simulink library "LibrarySimulinkSASv2014b"  (R2014b)
settings: Solver=ode45  SolverMode=SingleTasking  StartTime=0.0  StopTime=10.0  RelTol=1e-3
undefined symbols (not defined in the file or in its folder): shmControl, ts
undefined symbols (not defined in the file): IdB, IdL, IdM
libraries: LibrarySimulinkSAS x15, $bdroot x1
(part 1 of 4)
settings Model: CovSaveCumulativeToWorkspaceVar=on  Creator=dsantiago  LastModifiedBy=dds

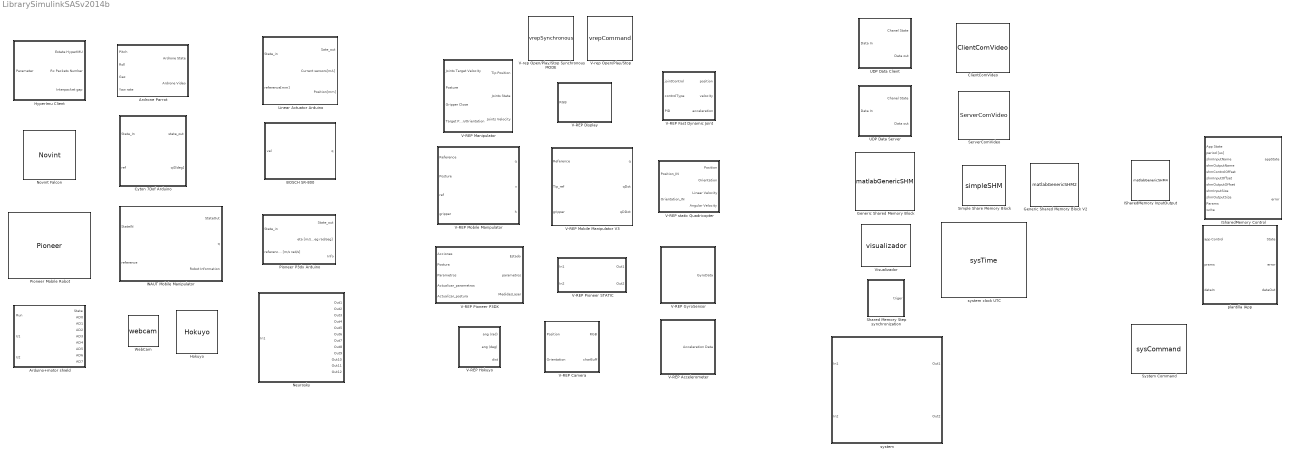
== system LibrarySimulinkSASv2014b ==
  Ardrone Parrot : Subsystem  mask{Dev='ARDRONE', ts=-1}
      mask prompts: Dev "Ardrone Parrot version"; ts "Sample Time"
      mask Help:
      | HELP DEVICE
      | 
      | - UDP Client for the server "ArdroneParrot.exe" App
      | 
      | HELP DRIVERS
      | 
      | -------------------------------------------------------------------------------------------------------------------------------------------------------
      | HELP APP
      | 
      | Before running the Simulink model initiate communication with the device through the application 'ardroneParrot.exe' available from folder '\SAS TOOLS\Software Ardrone Parrot V1.1.0'. After executing the program the console-type app is available.
      | 
      | 
      | -------------------------------------------------------------------------------------------------------------------------------------------------------
      | 
      | HELP BLOCK
      | 
      | Input Ports
      | 
      | Port 'Pitch reference': desde Matlab, entre -1 y 1, +-28.65 grados
      | Port 'Roll reference': desde Matlab, entre -1 y 1, +-28.65 grados
      | Port 'Gaz reference': desde Matlab, entre -1 y 1, +- 1 m/s
      | Port 'Yaw rate reference': desde Matlab, entre -1 y 1, +- 172 grados/segundo
      | 
      | Output Ports
      | 
      | Port 'State ArdroneParrot': sensors information from the Parrot quadcopter including a vector 'dispositivo' of 20 signals type doubles
      | 
      | dispositivo[0] -> Battery level (1 -> 100%)
      | dispositivo[1] -> Phi en rad (roll)
      | dispositivo[2] -> Theta en rad (pitch)
      | dispositivo[3] -> Psi en rad (yaw)
      | dispositivo[4] -> Altitud (en metros)
      | dispositivo[5] -> Velocidad eje X (forward) (en m/s)
      | dispositivo[6] -> Velocidad eje Y (sideward) (en m/s)
      | dispositivo[7] -> Velocidad eje Z (en m/s)
      | dispositivo[8] -> Giróscopo[0] (en deg/s)
      | dispositivo[9] -> Giróscopo[1] (en deg/s)
      | dispositivo[10] -> Giróscopo[2] (en deg/s)
      | dispositivo[11] -> Acelerómetro[0] (en mili g)
      | dispositivo[12] -> Acelerómetro[1] (en mili g)
      | dispositivo[13] -> Acelerómetro[2] (en mili g)
      | dispositivo[14] -> Latitud (en deg)
      | dispositivo[15] -> Longitud (en deg)
      | dispositivo[16] -> Elevación (en metros)
      | dispositivo[17] -> WIFI signal quality (?)
      | dispositivo[18] -> Boton 3 (Off = 0.0 - On = 1.0)
      | dispositivo[19] -> Boton 4 (Off = 0.0 - On = 1.0)
      | 
      | -------------------------------------------------------------------------------------------------------------------------------------------------------
      | 
      | HELP SHARE MEMORY (information only for external applications different to "ArdroneParrot.exe")
      | 
      | Shared Memory Names:
      | 
      | 'ARDRONE_entrada_'#dev
      | 'ARDRONE_salida_'#dev
      | NOTE: replace #dev with the device number
      mask Display:
      | image('parrot.png')
      | color('red')
      | port_label('input',1,'Pitch reference')
      | port_label('input',2,'Roll reference')
      | port_label('input',3,'Gaz reference')
      | port_label('input',4,'Yaw rate reference')
      | color('blue')
      | port_label('output',1,'Quadcopter state')
      | port_label('output',2,'Front Camera')
  Arduino+motor shield : Subsystem  mask{dev=1, ts=-1}
      mask prompts: dev "Device Number"; ts "Sample Time"
      mask Help:
      | HELP DEVICE
      | 
      | - App for using the kit arduino UNO plus Adafruit motor shield 1.2 based on two L293D chips forming 4 H-Bridges. L293D chipset provides 0.6A per bridge (1.2A peak) with thermal shutdown protection, internal kickback protection diodes. Can run motors on 4.5VDC to 25VDC. The shield is compatible with Arduino Mega 1280 & 2560, Diecimila, Duemilanove, and UNO
      | 
      | HELP DRIVERS
      | 
      | Upload the firmware 'Arduino_motor_firmware' in your Arduino UNO kit available from folder '\SAS TOOLS\Software arduino_motor V1.1.0'
      | -------------------------------------------------------------------------------------------------------------------------------------------------------
      | HELP APP
      | 
      | Before running the Simulink model initiate communication with the device through the application 'Arduino_motor.exe' available from folder '\SAS TOOLS\Software arduino_motor V1.1.0'. After executing the program the app icon is available in the taskbar.
      | The app "arduino_motor.exe" is handled from right-click on the icon and selecting the corresponding option:
      | "Play" -> Start the commnunication between the device and the app
      | "Stop" -> Stop the communication
      | "Monitor"->view the state of sensors and communication
      | 
      | -------------------------------------------------------------------------------------------------------------------------------------------------------
      | 
      | HELP BLOCK
      | 
      | Input Ports
      | 
      | Port 'Run': 1 for running and 0 for stopping
      | 
      | Port 'U1': value between [-255;255] which drives the tension applied to the motor 1 of the shield motor Adafruit 1.0
      | Port 'U2': value between [-255;255] which drives the tension applied to the motor 2 of the shield motor Adafruit 1.0
      | Port 'U3': value between [-255;255] which drives the tension applied to the motor 3 of the shield motor Adafruit 1.0
      | Port 'U4': value between [-255;255] which drives the tension applied to the motor 4 of the shield motor Adafruit 1.0
      | 
      | The range [-255;255] is related with the tension range [-V;V], where V is the tension source applied to the motor shield
      | 
      | Output Ports
      | 
      | Port 'State': information about the system. Run=1; Stop=0
      | 
      | Port 'AD0': value between [0;1023] measured in the pin AD0 of the Arduino UNO board
      | Port 'AD1': value between [0;1023] measured in the pin AD1 of the Arduino UNO board
      | Port 'AD2': value between [0;1023] measured in the pin AD2 of the Arduino UNO board
      | Port 'AD3': value between [0;1023] measured in the pin AD3 of the Arduino UNO board
      | 
      | The range [0;1023] is related with the tension range [0;5V] readed on the pins of the Arduino kit A/D conversor
      | -------------------------------------------------------------------------------------------------------------------------------------------------------
      | 
      | HELP SHARE MEMORY (information only for external applications different to "Arduino_motor.exe")
      | 
      | Shared Memory Names:
      | 
      | 'ARDUINO_entrada_'#dev
      | 'ARDUINO_salida_'#dev
      | NOTE: replace #dev with the device number
      mask DialogControl DescGroupVar Prompt: Arduino motor shield
      mask DialogControl DescTextVar Prompt: This block is used to receive and send data via USB port between a UNO Arduino board and the App "Arduino_motor.exe" available on folder '\SAS TOOLS\Software Arduino_motor V1.1.0'. You must upload the firmware "Arduino_motor_firmware" into your Arduino board. More information in Help button.
      mask Display:
      | image('motor_shield.png')
      | color('red')
      | port_label('input',1,'run')
      | port_label('input',2,'U1')
      | port_label('input',3,'U2')
      | port_label('input',4,'U3')
      | port_label('input',5,'U4')
      | color('blue')
      | port_label('output',1,'state')
      | port_label('output',2,'AD0')
      | port_label('output',3,'AD1')
      | port_label('output',4,'AD2')
      | port_label('output',5,'AD3')
  BOSCH SR-800 : Subsystem  mask{id=11}
      mask prompts: id "Id Memoria Compartida"
      mask Display:
      | image('bosch.jpg')
      | color('white');
      | port_label('input', 1, 'Velocidades')
      | port_label('output', 1, 'Pos. Articular')
  ClientComVideo : S-Function  FunctionName=ClientComVideo  SFunctionDeploymentMode=off  EnableBusSupport=off
      mask Help:
      | This block is used to transmit large data vectorsover the network. For small sizes use the block 'ClientCom'.
      | This block depends on the external program 'Cliente.exe' placed on '\SAS TOOLS\Software Network Communication V2.0.0\Video Transmission\Cliente'
      | After executing, the program is started minimized in the taskbar.
      | To initiate communication first configure the program : right click on the icon ->Configurar.
      | On section 'Configuracion de Ip y Puertos', load the input IP address and the output IP address of the Client adaptor. If there are multiple adaptors, these IP may be identical or different for the input and output data. Also select the ports for input and output.
      | On section 'Datos de Conexion con el Servidor', load the IP address and the input port of the Server.
      | On seccion 'Protocolo' select TCP or UDP. (The TCP option still in development)
      | 
      | Input Ports
      | 
      | The vector data to be transmitted must be introduced into the port 'dataSend'. Its maximun size is 60000 doubles.
      | The size of data to be transmitted must be specified on port 'sizeSend'.
      | The transmission occurs in each pulse on port 'Clock'. Use this port to set the transmission rate.
      | 
      | Output Ports
      | 
      | Port 'stateChanel'contains information concerning the transmission of data. For details of the composition of this vector right-click the icon of the taskbar -> 'Estado'
      | Port 'dataRecv' contains the data vector received from the server.
      | 
      | Shared Memory Structure:
      | 
      | typedef struct tag_NET_VIRTUAL_DEVICE_IN {
      | 	bool signalWrite;
      | 	UINT sizeMemoryOut;
      | } NET_VIRTUAL_DEVICE_IN;
      | 
      | typedef struct tag_NET_VIRTUAL_DEVICE_OUT {
      | 	bool signalRead;	//Se leen los flancos
      | 	UINT numberSentPackets;
      | 	UINT numberRecvPackets;
      | 	UINT numberLostPackets;
      | 	UINT numberDelPackets;
      | 	float rateSentPackets;
      | 	float rateRecvPackets;
      | 	float rateLostPackets;
      | 	float rateDelPackets;
      | 	float roundTripDelay;
      | 
      | 	UINT remoteNumberSentPackets;
      | 	UINT remoteNumberRecvPackets;
      | 	UINT remoteNumberLostPackets;
      | 	UINT remoteNumberDelPackets;
      | 	float remoteRateSentPackets;
      | 	float remoteRateRecvPackets;
      | 	float remoteRateLostPackets;
      | 	float remoteRateDelPackets;
      | 	float remoteRoundTripDelay;
      | } NET_VIRTUAL_DEVICE_OUT;
      | 
      | 
      | typedef struct tag_VIRTUAL_DEVICE_IN {
      | 	float dataMemory[60000];
      | } VIRTUAL_DEVICE_IN;
      | 
      | typedef struct tag_VIRTUAL_DEVICE_OUT {
      | 	float dataMemory[60000];
      | } VIRTUAL_DEVICE_OUT;
      mask DialogControl DescGroupVar Prompt: Client data transmission through the network
      mask DialogControl DescTextVar Prompt: This block is used to exchange large amount of data (video) over a network. Use this block client-side communication.
      mask Display:
      | image('client.png')
      | color('red')
      | port_label('input',1,'clock')
      | port_label('input',2,'sizeSend')
      | port_label('input',3,'dataSend')
      | color('blue')
      | port_label('output',1,'stateChannel')
      | port_label('output',2,'sizeMustBeRecv')
      | port_label('output',3,'dataRecv')
  Cyton 7Dof Arduino : Subsystem  mask{id=IdM, ts=ts}
      mask prompts: id "Device Number"; ts "Sample Time"
      mask Display:
      | image('cytonArm.png')
      | color('red')
      | port_label('input',1,'State')
      | port_label('input',2,'reference[?]')
      | 
      | color('blue')
      | port_label('output',1,'State')
      | port_label('output',2,'qi[ºdeg]')
  Generic Shared Memory Block : S-Function  FunctionName=matlabGenericSHM  Parameters=nombre,dispositivo,vecE,vecS,tipoE,tipoS,ts  SFunctionDeploymentMode=off  EnableBusSupport=off  mask(Generic Share Memory block V2.0){nombre='memoriaEjemplo', dispositivo=10, vecE=[3 2 1], vecS=[4 5 6 156], tipoE=['f' 'I' 'c'], tipoS=['C' 'i' 'd' 'd'], ts=-1}
      mask prompts: nombre "Share Memory base name"; dispositivo "Device Number"; vecE "Sizes input vector"; vecS "Sizes output vector"; tipoE "Data Types Inputs"; tipoS "Data Types Outputs"; ts "Sample Time"
      mask help:
      | This block allows Simulink communication with external processes through shared memory. It works on Unix and Windows.
      | More information in Help.
      mask Help:
      | Share Memory base name (nombre): It's the base string of share memory ej 'robot'
      | Device Number (dispositivo): integer that identifies the device between multiple in use
      | Two shared memorys are created with names:
      | "%name_entrada_%dispositivo" for the device input (writing)
      | "%name_salida_%dispositivo" for the device output (reading)
      | Sizes input vector (vecE)  : array that specify the number of inputs (array dimension) and the inputs size (array values)
      | Sizes output vector  (vecS): array that specify the number of outputs (array dimension) and the outputs size (array values)
      | Data types input(tipoE): char array that specify each input types
      | Data types output (tipoS): char array that specify each output types
      | Allowed types are:
      | ('f'=float, 'd'=double, 'i'=int32, 'c'=char, 'C'= unsigned char, 'I'=unsigned int)
      | Example.
      | Suppose the following parameters:
      | nombre='exampleMemory'
      | dispositivo=10
      | vecE=[3 2 1]
      | vecS=[6 5 7 8]
      | tipoE=['f' 'I' 'c']
      | tipoS=['C' 'i' 'd' 'd']
      | 
      | Two Share Memories are created with names:
      | "exampleMemory_entrada_10" (block input)
      | "exampleMemory_salida_10"  (block output)
      | 3 inputs are created:
      |  1° Dimension 3, type "unsigned char"
      |  2° Dimension 2, type "int"
      |  3° Dimension 1, type "char"
      | 4 outputs are created:
      |  1° Dimension 6, type "float"
      |  2° Dimension 5, type "unsigned int"
      |  3° Dimension 7, type "double"
      |  3° Dimension 8, type "double"
      mask Display: image('shmem.jpg')
  Generic Shared Memory Block V2 : S-Function  FunctionName=matlabGenericSHM2  Parameters=devInMem,devOutMem,vecE,vecS,tipoE,tipoS,ts  SFunctionDeploymentMode=off  EnableBusSupport=off  mask(Generic Share Memory block V2.0){devInMem='device_actions', devOutMem='device_sensors', vecE=[3 2 1], vecS=[4 5], tipoE=['f' 'I' 'c'], tipoS=['C' 'f'], ts=-1}
      mask prompts: devInMem "Name of the input memory of the device"; devOutMem "Name of the output memory of the device"; vecE "Sizes input vector"; vecS "Sizes output vector"; tipoE "Data Types Inputs"; tipoS "Data Types Outputs"; ts "Sample Time"
      mask help:
      | This block allows Simulink communication with external processes through shared memory. It works on Unix and Windows.
      | More information in Help.
      mask Help:
      | Name of reading Shared Memory: It is the name of the memory that Simulink uses to update the output of the block. In your external application write the states of the device in this memory.
      | Name of writing Shared Memory: It is the name of the memory in which Simulink writes the entries to the block. In its external application read this memory to obtain the commands of the device.
      | Sizes input vector (vecE)  : array that specify the number of inputs (array dimension) and the inputs size (array values)
      | Sizes output vector  (vecS): array that specify the number of outputs (array dimension) and the outputs size (array values)
      | Data types input(tipoE): char array that specify each input types
      | Data types output (tipoS): char array that specify each output types
      | Allowed types are:
      | ('f'=float, 'd'=double, 'i'=int32, 'c'=char, 'C'= unsigned char, 'I'=unsigned int)
      | 
      | Example.
      | Suppose the following parameters:
      | Name of reading Shared Memory:device_sensors
      | Name of writing Shared Memory:device_actuators
      | vecE=[3 2 1]
      | vecS=[6 5 7 8]
      | tipoE=['f' 'I' 'c']
      | tipoS=['C' 'i' 'd' 'd']
      | 
      | Two Share Memories are created with names:
      | "device_sensors" (block input)
      | "device_actuators"  (block output)
      | 3 inputs are created:
      |  1° Dimension 3, type "unsigned char"
      |  2° Dimension 2, type "int"
      |  3° Dimension 1, type "char"
      | 4 outputs are created:
      |  1° Dimension 6, type "float"
      |  2° Dimension 5, type "unsigned int"
      |  3° Dimension 7, type "double"
      |  3° Dimension 8, type "double"
      mask Display: image('mem2.png')
  Hokuyo : S-Function  FunctionName=Hokuyo  SFunctionDeploymentMode=off  EnableBusSupport=off
      mask Display:
      | image('hokuyo.jpg')
      | color('red');
      | port_label('output', 1, 'Angle[rad]')
      | port_label('output', 2, 'Angle[deg]')
      | port_label('output', 3, 'Distance[m]')
  HyperImu Client : Subsystem  mask{dev=1, ts=-1}
      mask prompts: dev "Device Number"; ts "Sample Time"
      mask Help:
      | HELP DEVICE
      | 
      | - UDP Client for the server "HyperIMU"-Android App
      | 
      | HELP DRIVERS
      | 
      | Install in your phone the app "HyperIMU" for Android. Link: https://play.google.com/store/apps/details?id=com.ianovir.hyper_imu&hl=es
      | -------------------------------------------------------------------------------------------------------------------------------------------------------
      | HELP APP
      | 
      | Before running the Simulink model initiate communication with the device through the application 'imu.exe' available from folder '\SAS TOOLS\Software imu V1.1.0'. After executing the program the app icon is available in the taskbar.
      | The app "HyperIMU.exe" is handled from right-click on the icon and selecting the corresponding option:
      | "Play" -> Start the commnunication between the device and the app
      | "Stop" -> Stop the communication
      | "Monitor"->view the state of sensors and communication
      | 
      | -------------------------------------------------------------------------------------------------------------------------------------------------------
      | 
      | HELP BLOCK
      | 
      | Input Ports
      | 
      | Port 'Parameters' scalar non-used.
      | 
      | Output Ports
      | 
      | Port 'State': sensors information chosen with the App HyperIMU
      | 
      | 'Rx Packets Number' number of received packets by the client
      | 
      | 'Interpacket gap' time between packets
      | 
      | -------------------------------------------------------------------------------------------------------------------------------------------------------
      | 
      | HELP SHARE MEMORY (information only for external applications different to "HyperIMU.exe")
      | 
      | Shared Memory Names:
      | 
      | 'HYPERIMU_entrada_'#dev
      | 'HYPERIMU_salida_'#dev
      | NOTE: replace #dev with the device number
      mask DialogControl DescGroupVar Prompt: HyperIMU client
      mask DialogControl DescTextVar Prompt: This block is used to receive data via UDP from the App "HyperIMU" through the application 'HyperIMU.exe' available on folder '\SAS TOOLS\Software HyperIMU V1.1.0'. More information in Help button.
      mask Display:
      | image('HyperIMU.png')
      | color('red')
      | port_label('input',1,'Parameter')
      | 
      | color('blue')
      | port_label('output',1,'State HyperIMU')
      | port_label('output',2,'Rx Packets Number')
      | port_label('output',3,'Interpacket gap')
  INAUT Mobile Manipulator : Subsystem  mask{IdB=3, IdM=1, IdL=0, ts=-1}
      mask prompts: IdB "Mobile Base Id"; IdM "Manipulator Robot Id"; IdL "Linear Actuator Id"; ts "Sample Time"
      mask Display:
      | image('INAUT_mobile_manipulator.png')
      | color('red')
      | port_label('input',1,'State')
      | port_label('input',2,'Reference')
      | color('blue')
      | port_label('output',1,'State')
      | port_label('output',2,'q')
      | port_label('output',3,'Info')
  ISharedMemory Control : Subsystem  mask{shmControl=shmControl}
      mask prompts: shmControl "Control Name"
      mask Display:
      | image('mem4.png')
      | color('blue');
      | port_label('input', 1, 'App Control')
      | port_label('input', 2, 'Period[us]')
      | port_label('input', 3, 'Input Name')
      | port_label('input', 4, 'Output Name')
      | port_label('input', 5,'Control memory offset')
      | port_label('input', 6,'Input memory offset')
      | port_label('input', 7,'Output memory offset')
      | port_label('input', 8, 'Input Size')
      | port_label('input', 9, 'Output Size')
      | port_label('input', 10, 'Prams')
      | port_label('input', 11, 'Write to mem')
      | color('red');
      | port_label('output', 1, 'App ctr State')
      | port_label('output', 2, 'Error')
  ISharedMemory InputOutput : S-Function  FunctionName=matlabGenericSHM4  Parameters=nameIn,nameOut,vecE,vecS,tipoE,tipoS,ts,trigg,inOff,outOff  SFunctionDeploymentMode=off  EnableBusSupport=off  mask(ISharedMemory InputOutput (Generic Share Memory block V3.0)){nameIn='device_input', nameOut='device_output', vecE=[1 2], vecS=[3 4], tipoE=['f' 'd'], tipoS=['c' 'i'], inOff=10, outOff=20, ts=-1, trigg=on}
      mask prompts: nameIn "Input Shared Memory Name"; nameOut "Output Shared Memory Name"; vecE "Vector of system inputs"; vecS "Vector of system outputs"; tipoE "Data Types Inputs"; tipoS "Data Types Outputs"; inOff "Input Shared Memory Offset"; outOff "Output Shared Memory Offset"; ts "Sample Time"; trigg "Triggered writing"
      mask help:
      | This block allows Simulink communication with external processes through shared memory. It works on Unix and Windows.
      | More information in Help.
      mask Help:
      | Input Shared Memory Name: Name of the memory from which the physical device acquires its inputs.
      | Output Shared Memory Name: Name of the memory in which the physical device writes its output data.
      | Vector of system inputs: array that specify the number of inputs (array dimension) and the inputs size (array values)
      | Vector of system outputs: array that specify the number of outputs (array dimension) and the outputs size (array values)
      | Data types input: char array that specify each input types
      | Data types output: char array that specify each output types
      | Allowed types are:
      | ('f'=float, 'd'=double, 'i'=int32, 'c'=char, 'C'= unsigned char, 'I'=unsigned int)
      | 
      | Imput Shared Memory Offset: Position (in bytes) from which data is written into the input memory to the device.
      | Output Shared Memory Offset: Position (in bytes) from which the data from the output memory of the device is read.
      | Note: the final size of the memory is equal to the amount of I/O multiplied by the size of each I/O plus the offset. For example, a memory with size=[2 3] , type =['f' 'c'] and offset = 10 will have a final size of 2 * 4 + 3 * 1 + 10 = 21 bytes, although only the 2*4 +3*1 = 11 last bytes are used.
      | 
      | Triggered writing: If trigger writing is selected, an additional block input is created at the end of inputs. When this input has a value greater than zero the data at the input of the block is written to the device input shared memory, otherwise the data is not written.
      | 
      | Sample Time: Explicit block sampling time.
      | 
      | Example.
      | Suppose the following parameters:
      | Input Shared Memory Name = "devIn_1"
      | Output Shared Memory Name = "devOut_1"
      | Vector of system inputs=[3 2 1]
      | Vector of system outputs=[6 5 7 8]
      | Data types output=['f' 'I' 'c']
      | tipoS=['C' 'i' 'd' 'd']
      | Imput Shared Memory Offset = 10
      | Output Shared Memory Offset =0
      | 
      | Two Share Memories are created with names:
      | "devIn_1"(block input)
      | "devOut_1"  (block output)
      | 3 inputs are created:
      |  1° Dimension 3, type "unsigned char"
      |  2° Dimension 2, type "int"
      |  3° Dimension 1, type "char"
      | 4 outputs are created:
      |  1° Dimension 6, type "float"
      |  2° Dimension 5, type "unsigned int"
      |  3° Dimension 7, type "double"
      |  3° Dimension 8, type "double"
      mask Display: image('mem4.png')
  Linear Actuator Arduino : Subsystem  mask{id=IdL, ts=ts}
      mask prompts: id "Device Number"; ts "Sample Time"
      mask Help:
      | HELP DEVICE
      | 
      | - App for using the kit arduino MEGA plus Mosnter motor shield V12 based on VNH2SP30 chip forming a H-Bridges. VNH2SP30 chipset provides 20A per bridge (30A peak) with thermal shutdown protection, internal kickback protection diodes. Can run motors on 6VDC to 41VDC. The shield is compatible with Arduino Mega 1280 & 2560, Diecimila, Duemilanove, and UNO
      | 
      | HELP DRIVERS
      | 
      | Upload the firmware 'Firmware_Actuador' in your Arduino MEGA kit available from folder '\SAS TOOLS\Software arduino_actuador V1.1.0'
      | -------------------------------------------------------------------------------------------------------------------------------------------------------
      | HELP APP
      | 
      | First you have to uploadin the program 'Firmware_Actuador' to the Arduino MEGA available from folder '\SAS TOOLS\Software arduino_actuador V1.1.0'.
      | Before running the Simulink model initiate communication with the device through the application 'ArduinoSerie.exe' available from folder '\SAS TOOLS\Software arduino_actuador V1.1.0'. After executing the program the app icon is available in the taskbar.
      | The app "arduino_motor.exe" is handled from right-click on the icon and selecting the corresponding option:
      | "Play" -> Start the commnunication between the device and the app
      | "Stop" -> Stop the communication
      | "Monitor"->view the state of sensors and communication
      | 
      | -------------------------------------------------------------------------------------------------------------------------------------------------------
      | 
      | HELP BLOCK
      | 
      | Input Ports
      | 
      | Port 'Run': 1 for stop, 3 for start and 5 for pause
      | 
      | Port 'U1': value between [0;200] which drives the position of the actuator
      | 
      | The range [0;200] is related with the position range [0;mm], where mm is the extension that the actuator have
      | 
      | Output Ports
      | 
      | Port 'State': information about the system. Run=1; Stop=0
      | 
      | Port 'AD0': value between [0;20000] measured the current of the sensor 1
      | Port 'AD1': value between [0;20000] measured the current of the sensor 2
      | Port 'AD2': value between [0;20000] measured the current of the sensor 3
      | Port 'AD3': value between [0;20000] measured the real position of the actuator
      | 
      | The range [0;20000] is related with the tension range [0;5V] readed on the pins of the Arduino kit A/D conversor
      | -------------------------------------------------------------------------------------------------------------------------------------------------------
      | 
      | HELP SHARE MEMORY (information only for external applications different to "ArduinoSerie.exe")
      | 
      | Shared Memory Names:
      | 
      | 'ARDUINO_entrada_'#dev
      | 'ARDUINO_salida_'#dev
      | NOTE: replace #dev with the device number
      mask Display:
      | image('linearActuator.png')
      | color('red')
      | port_label('input',1,'State')
      | port_label('input',2,'Ref [mm]')
      | color('blue')
      | port_label('output',1,'State')
      | port_label('output',2,'Current Sensor[mA]')
      | port_label('output',3,'Position [mm]')
  Neurosky : Subsystem  mask{id=1, ts=-1}
      mask prompts: id "Device Id"; ts "Sample Time"
      mask DialogControl DescGroupVar Prompt: Neurosky device
      mask Display:
      | image('neurosky.jpg')
      | color('red')
      | port_label('input',1,'Start/Stop')
      | color('blue')
      | port_label('output',1,'POOR_SIGNAL')
      | port_label('output',2,'ATTENTION')
      | port_label('output',3,'MEDITATION')
      | port_label('output',4,'DATA RAW')
      | port_label('output',5,'DELTA')
      | port_label('output',6,'THETA')
      | port_label('output',7,'ALPHA1')
      | port_label('output',8,'ALPHA2')
      | port_label('output',9,'BETA1')
      | port_label('output',10,'BETA2')
      | port_label('output',11,'GAMMA1')
      | port_label('output',12,'GAMMA2')
  Novint Falcon : S-Function  FunctionName=Novint  Parameters=dev  SFunctionDeploymentMode=off  EnableBusSupport=off  mask{dev=1}
      mask prompts: dev "Device Number"
      mask Help:
      | HELP DEVICE
      | 
      | - Josytick 3D with force feedback "Novint Falcon". More information on http://www.novint.com/index.php/products/novintfalcon
      | 
      | HELP DRIVERS
      | 
      | Install the driver located in "SAS TOOLS\Software Novint V1.1.0\Driver"
      | 
      | -------------------------------------------------------------------------------------------------------------------------------------------------------
      | HELP APP
      | 
      | Before running the Simulink model initiate communication with the device through the application 'Novint.exe' available from folder '\SAS TOOLS\Software Novint V1.1.0'. After executing the program the app icon is available in the taskbar.
      | The app "Novint.exe" is handled from right-click on the icon and selecting the corresponding option:
      | "Play" -> Start the commnunication between the device and the app each 1 ms (sampling time)
      | "Stop" -> Stop the communication
      | "Monitor"->view the state of sensors and communication
      | "Config"-> configure the app: select device number and set the control parameters
      | 
      | -------------------------------------------------------------------------------------------------------------------------------------------------------
      | 
      | HELP BLOCK
      | 
      | Input Ports
      | 
      | Port 'Acciones' are the command imput of the robot composed of X,Y,Z force in [N]. Range [0-10] Newtons
      | Port 'Parametros' it is use to load the robot internal parameters wich are Spring Components (N/cm) in X,Y,Z axis and Damping component (N.s/cm)in X,Y,Z axis.
      | Port 'ActualizarParametros' it is used to update by rising or falling edge the parameters loaded in port 'Parametros'
      | 
      | Output Ports
      | 
      | Port 'Estado' contains the position in [cm] (X,Y,Z) and velocities in [cm/s] (X,Y,Z) of the end effector. Worskpace [-5;5] cm in each axis
      | Port 'Botones' contains the states of the buttons on the end effector.
      | 
      | -------------------------------------------------------------------------------------------------------------------------------------------------------
      | 
      | HELP FIRMWARE
      | 
      | 
      | --------------------------------------------------------------------------------------------------------------------------------------------------
      | 
      | HELP SHARE MEMORY (information only for external applications different to "Novint.exe")
      | 
      | Shared Memory Names:
      | 
      | 'novint_entrada_'#dev
      | 'novint_salida_'#dev
      | NOTE: replace #dev with the device number
      | The Share Memory Estructure is:
      | 
      | typedef struct tag_VIRTUAL_DEVICE_ENTRADA {
      | 	double Acciones[3];
      | 	double Parametros[6];
      | 	bool Actualizar_parametros;
      | } VIRTUAL_DEVICE_ENTRADA;
      | 
      | typedef struct tag_VIRTUAL_DEVICE_SALIDA {
      | 	double Estado[6];
      | 	bool Botones[4];
      | } VIRTUAL_DEVICE_SALIDA;
      | 
      | --------------------------------------------------------------------------------------------------------------------------------------------------------------------
      mask DialogControl DescTextVar Prompt: This block is used to command the Novint Falcon Manipulator through the application 'Novint.exe' available on folder '\SAS TOOLS\Software Novint V1.1.0'. More information in Help button.
      mask DialogControl Control2 Prompt: For multiple devices, set diferent number for each one.
      mask Display:
      | image('novint.jpg')
      | color('red')
      | port_label('input',1,'Acciones')
      | port_label('input',2,'Parametros')
      | port_label('input',3,'ActualizarParametros')
      | color('blue')
      | port_label('output',1,'Estado')
      | port_label('output',2,'Botones')
  "Pioneer Mobile Robot " : S-Function  FunctionName=Pioneer  Parameters=dev  SFunctionDeploymentMode=off  EnableBusSupport=off  mask{dev=0}
      mask prompts: dev "Device Number"
      mask Help:
      | External dependencies
      | 
      | Before running the Simulink application initiate communication with the robot pioneer through the application 'Pioneer V1.0.0.exe' available from the '\ SAS TOOLS \ Software V1.0.0 Pioneer' folder. After executing the program is started minimized in the taskbar.
      | To initiate communication right-click on the icon -> Enable.
      | 
      | Input Ports
      | 
      | Port 'Acciones' are the command imput of the robot composed of linear and angular velocities.
      | Port 'Postura' it is used to load the initial posture af the robot for the internal odometry
      | Port 'ActualizarPostura' it is used to set the parameters loded in port 'Postura'
      | Port 'Parametros' it is use to load the robot internal PID controller
      | Port 'ActualizarParametros' it is used to set the parameters loded in port 'Parametros'
      | 
      | Output Ports
      | 
      | Port 'Estados' contains odometry information in its first three components and the robot linear and angular velocity in its last two components.
      | Port 'Parametros' contains the current parameters set in the robot.
      | Port 'Laser ' contains the Laser dots information.
      | 
      | The Share Memory Estructure is:
      | 
      | typedef struct DEVICE_INPUT {
      | 	double Acciones[2];
      | 	double Postura[3];
      | 	int Parametros[6];
      | 	bool Actualizar_parametros;
      | 	bool Actualizar_postura;
      | } VIRTUAL_INPUT;
      | 
      | DEVICE_OUTPUT {
      | 	double Estado[5];
      | 	int Parametros[6];
      | 	unsigned int MedidasLaser[181];
      | } VIRTUAL_OUTPUT;
      mask DialogControl DescGroupVar Prompt: Pioneer Mobile Robots
      mask DialogControl DescTextVar Prompt: This block is used to command the Pioneer mobile robot , through the application 'Pioneer V1.0.0' available on folder 'SAS TOOLS \ Software Pioneer V1.0.0'. More information in Help button.
      mask DialogControl Container2 Prompt: Parameters
      mask Display:
      | image('pioneer3dx.png')
      | color('red')
      | port_label('input',1,'Acciones')
      | port_label('input',2,'Postura')
      | port_label('input',3,'Parametros')
      | port_label('input',4,'ActualizarParametros')
      | port_label('input',5,'ActualizarPostura')
      | color('blue')
      | port_label('output',1,'estado')
      | port_label('output',2,'parametros')
      | port_label('output',3,'laser')
  Pioneer P3dx Arduino : Subsystem  mask{id=IdB, ts=ts}
      mask prompts: id "Device Id"; ts "Sample Time"
      mask Display:
      | image('pioneerp2dx.jpg')
      | color('red')
      | port_label('input',1,'State')
      | port_label('input',2,'Reference')
      | color('blue')
      | port_label('output',1,'State')
      | port_label('output',2,'eta')
      | port_label('output',3,'Info')
  ServerComVideo : S-Function  FunctionName=ServerComVideo  SFunctionDeploymentMode=off  EnableBusSupport=off
      mask Help:
      | This block is used to transmit large data vectors. For small sizes use the block 'ServerCom'.
      | This block depends on the external program 'Servidor.exe' placed on '\SAS TOOLS\Software Network Communication V2.0.0\Video Transmission\Servidor'
      | After executing, the program is started minimized in the taskbar.
      | To initiate communication first configure the program : right click on the icon ->Configurar.
      | On section 'Configuracion de Ip y Puertos', load the input IP address and the output IP address of the computer adaptor. If there are multiple adaptors, these IP may be identical or different for the input and output data. Also select the ports for input and output.
      | On seccion 'Protocolo' select TCP or UDP. (The TCP option still in development)
      | 
      | Input Ports
      | 
      | The vector data to be transmitted must be introduced into the port 'dataSend'. Its maximun size is 60000 elements.
      | The size of data to be transmitted must be specified on port 'sizeSend'.
      | The transmission occurs in each pulse on port 'Clock'. Use this port to set the transmission rate.
      | 
      | Output Ports
      | 
      | Port 'stateChanel'contains information concerning the transmission of data. For details of the composition of this vector right-click the icon of the taskbar -> 'Estado'
      | Port 'dataRecv' contains the data vector received from the server.
      | 
      | 
      | Shared Memory Structure:
      | 
      | typedef struct tag_NET_VIRTUAL_DEVICE_IN {
      | bool signalWrite;
      | UINT sizeMemoryOut;
      | } NET_VIRTUAL_DEVICE_IN;
      | 
      | typedef struct tag_NET_VIRTUAL_DEVICE_OUT {
      | bool signalRead;	//Se leen los flancos
      | UINT numberSentPackets;
      | UINT numberRecvPackets;
      | UINT numberLostPackets;
      | UINT numberDelPackets;
      | float rateSentPackets;
      | float rateRecvPackets;
      | float rateLostPackets;
      | float rateDelPackets;
      | float roundTripDelay;
      | 
      | UINT remoteNumberSentPackets;
      | UINT remoteNumberRecvPackets;
      | UINT remoteNumberLostPackets;
      | UINT remoteNumberDelPackets;
      | float remoteRateSentPackets;
      | float remoteRateRecvPackets;
      | float remoteRateLostPackets;
      | float remoteRateDelPackets;
      | float remoteRoundTripDelay;
      | } NET_VIRTUAL_DEVICE_OUT;
      | 
      | 
      | typedef struct tag_VIRTUAL_DEVICE_IN {
      | float dataMemory[60000];
      | } VIRTUAL_DEVICE_IN;
      | 
      | typedef struct tag_VIRTUAL_DEVICE_OUT {
      | float dataMemory[60000];
      | } VIRTUAL_DEVICE_OUT;
      mask DialogControl DescGroupVar Prompt: Server data transmission through the network
      mask DialogControl DescTextVar Prompt: This block is used to exchange large amount of data over a network. Use this block server-side communication.
      mask Display:
      | image('server.png')
      | color('red')
      | port_label('input',1,'clock')
      | port_label('input',2,'sizeSend')
      | port_label('input',3,'dataSend')
      | color('blue')
      | port_label('output',1,'stateChannel')
      | port_label('output',2,'sizeMustBeRecv')
      | port_label('output',3,'dataRecv')
  Shared Memory Step synchronization : Subsystem  mask(Shared Memory synchronization block){id=5}
      mask prompts: id "Memory Id"
      mask help: When a value of the shared memory change, a pulse is generated on output Trigger.
      mask Help:
      | When a value of the shared memory change, a pulse is generated on output Trigger.
      | On server side (hardware or simulator) implement a counter and change its value on each step. Store the counter value on "'sync_counter'"_id shared memory
      mask Display:
      | image('sync.png')
      | color('blue')
      | port_label('output',1,'trigger')
  Simple Share Memory Block : S-Function  FunctionName=simpleSHM  Parameters=nombre,dispositivo,vecE,vecS,tipoE,tipoS  SFunctionDeploymentMode=off  EnableBusSupport=off  mask(Share Memory Block version 2.0){nombre='memoriaEjemplo', dispositivo=10, vecE=[3 2 1], vecS=[], tipoE=['f' 'I' 'c'], tipoS=[]}
      mask prompts: nombre "Share Memory base name"; dispositivo "Device Number"; vecE "Sizes input vector"; vecS "Sizes output vector"; tipoE "Data Types Inputs"; tipoS "Data Types Outputs"
      mask help: This block allows Simulink communication with external processes through shared memory. It works on Unix and Windows
      mask Help:
      | Share Memory base name (nombre): It's the base string of share memory ej 'robot'
      | Device Number (dispositivo): integer that identifies the device between multiple in use
      | Only one Share memory will be created, so you should specify only the inputs or outputs but not both
      | One shared memorys is created with name: "%name_%dispositivo"
      | 
      | Sizes input vector (vecE)  : array that specify the number of inputs (array dimension) and the inputs size (array values)
      | Sizes output vector  (vecS): array that specify the number of outputs (array dimension) and the outputs size (array values)
      | Data types input(tipoE): char array that specify each input types
      | Data types output (tipoS): char array that specify each output types
      | Allowed types are:
      | ('f'=float, 'd'=double, 'i'=int32, 'c'=char, 'C'= unsigned char, 'I'=unsigned int)
      | 
      | Example.
      | Suppose the following parameters:
      | nombre='exampleMemory'
      | dispositivo=10
      | vecE=[3 2 1]
      | vecS=[]
      | tipoE=['f' 'I' 'c']
      | tipoS=[]
      | 
      | One Share Memorie is created with name:
      | "exampleMemory_10" (block input)
      | 
      | 3 inputs are created:
      |  1° Dimension 3, type "unsigned char"
      |  2° Dimension 2, type "int"
      |  3° Dimension 1, type "char"
      mask Display: image('memoryChip.png')
  System Command : S-Function  FunctionName=sysCommand  Parameters=start,update,outputs,terminate  SFunctionDeploymentMode=off  EnableBusSupport=off  mask{start='start vrep.exe & timeout /t 10', update='', outputs='', terminate='taskkill /IM vrep.exe'}
      mask prompts: start "On Start() Command"; update "On Update() command"; outputs "On Output() command"; terminate "On Terminate() command"
      mask Display: image('syscmd.png')
  UDP Data Client : Subsystem  mask{ts=.1, n=5}
      mask prompts: ts "Sample Time"; n "Output Array Size (max 1024)"
      mask DialogControl DescTextVar Prompt: Client for UDP Data Server Block
      mask Display:
      | image('client.png')
      | color('red')
      | port_label('input',1,'Data Send')
      | color('blue')
      | port_label('output',1,'stateChannel')
      | port_label('output',2,'Data Receive')
  UDP Data Server : Subsystem  mask{ts=.05, n=8}
      mask prompts: ts "Sample Time"; n "Output Array Size (max 1024)"
      mask Display:
      | image('client.png')
      | color('red')
      | port_label('input',1,'Data Send')
      | color('blue')
      | port_label('output',1,'stateChannel')
      | port_label('output',2,'Data Receive')
  V-REP Accelerometer : Subsystem  mask{n=3}
      mask prompts: n "Device Number"
      mask Display:
      | image('accel.png')
      | color('red')
      | port_label('output',1,'Linear Acceleration')
  V-REP Camera : Subsystem  mask{ResX=512, ResY=512, id=4}
      mask prompts: ResX "Resolucion Camara X"; ResY "Resolucion Camara Y"; id "Id Memoria Compartida"
      mask Display:
      | image('webcam.jpg')
      | color('red');
      | port_label('input',1,'Posicion')
      | port_label('input',2,'Orientacion')
      | port_label('output',1,'RGB')
      | port_label('output',2,'Char Buffer')
  V-REP Display : Subsystem  mask{ResX=1080, ResY=1920, id=11, ts=-1}
      mask prompts: ResX "Resolucion Camara X"; ResY "Resolucion Camara Y"; id "Id Memoria Compartida"; ts "Sample Time"
      mask Display:
      | image('display.jpg')
      | color('white');
      | port_label('input',1,'RGB')
      | port_label('input',2,'Retardo')
  V-REP Fast Dynamic Joint : Subsystem  mask{id=1}
      mask prompts: id "DeviceNumber"
      mask Display:
      | color('red');
      | port_label('output',1,'Position');
      | port_label('output',2,'Velocity');
      | port_label('output',3,'Acceleration');
      | color('blue')
      | port_label('input',1,'Control Signal');
      | port_label('input',2,'Control Type');
      | port_label('input',3,'PID Paras');
  V-REP GyroSensor : Subsystem  mask{n=4}
      mask prompts: n "Device Number"
      mask Display:
      | image('euler.png')
      | color('red')
      | port_label('output',1,'Euler Angles')
  V-REP Hokuyo : Subsystem  mask{id=3, ts=-1}
      mask prompts: id "Id Memoria Compartida"; ts "Sample Time"
      mask Display:
      | image('hokuyo_vrep.png')
      | color('red');
      | port_label('output', 1, 'Angle[rad]')
      | port_label('output', 2, 'Angle[deg]')
      | port_label('output', 3, 'Distance[m]')
  V-REP Manipulator : Subsystem  mask{id=2, ts=-1}
      mask prompts: ts "Sample Time"
      mask Display:
      | color('blue');
      | image('phx.jpg')
      | port_label('input', 1, 'Joints Command')
      | port_label('input', 2, 'Posture')
      | port_label('input', 3, 'Gripper Close')
      | port_label('input', 4, 'Target')
      | color('red');
      | port_label('output', 1, 'Tip Position')
      | port_label('output', 2, 'Joints Position')
      | port_label('output', 3, 'Joints Velocity')
  V-REP Mobile Manipulator : Subsystem  mask{id1=1, id2=2}
      mask prompts: id1 "Id Memoria Compartida Pioneer"; id2 "Id Memoria Compartida Manipulador"
      mask Display:
      | image(imread('vrepMM.jpg'))
      | 
      | color('green');
      | port_label('input', 1, 'v(t)/tau(t)')
      | port_label('input', 2, 'Posture')
      | port_label('input', 3, 'tip_ref')
      | port_label('input', 4, 'gripper')
      | color('red');
      | port_label('output', 1, 'q (t)')
      | port_label('output', 2, 'v (t)')
      | port_label('output', 3, 'h (t)')
  V-REP Mobile Manipulator V3 : Subsystem  mask{id=1}
      mask prompts: id "Shared memory identifier (id)"
      mask help:
      | Shared Memory Identifiers:
      | id+1 : Mobile Base Right Weel Joint
      | id+2 : Mobile Base Left Weel Joint
      | id+1 : Arm Robot Joint 1
      | id+2 : Arm Robot Joint 2
      | id+3 : Arm Robot Joint 3
      | id+4 : Arm Robot Joint 4
      mask Display:
      | image(imread('vrepMM.jpg'))
      | 
      | color('green');
      | port_label('input', 1, 'reference')
      | port_label('input', 2, 'tip_ref')
      | port_label('input', 3, 'gripper')
      | color('red');
      | port_label('output', 1, 'q')
      | port_label('output', 2, 'qDot')
      | port_label('output', 3, 'qDDot')
  V-REP Pioneer P3DX : Subsystem  mask{id=1, ts=0}
      mask prompts: id "Device Number"; ts "Sample Time"
      mask Help:
      | External dependencies
      | 
      | Before running the Simulink application initiate the simulation on VREP.
      | 
      | Input Ports
      | 
      | The port 'Actions' transmits control actions to the simulated model. The nature of the control input control type depends on the model set VREP. These inputs may be Forces [N], Speed [m/s; rad/s] or references to the local PID speed controller [m/s; rad/s].
      | Port 'Postura' it is used to load the desired robot posture in the scene.
      | Port 'ActualizarPostura' it is used to set the the posture loded on port 'Postura' in the VREP scene
      | Port 'Parametros' it is use to load the robot internal PID controller
      | Port 'ActualizarParametros' it is used to set the parameters loded in port 'Parametros'
      | 
      | Output Ports
      | 
      | Port 'Estados' contains odometry information in its first three components and the robot linear and angular velocity in its last two components.
      | Port 'Parametros' contains the current parameters set in the robot.
      | Port 'Laser ' contains the Laser dots information.
      | 
      | The Share Memory Estructure is:
      | 
      | typedef struct DEVICE_INPUT {
      | double Acciones[2];
      | double Postura[3];
      | int Parametros[6];
      | bool Actualizar_parametros;
      | bool Actualizar_postura;
      | } VIRTUAL_INPUT;
      | 
      | DEVICE_OUTPUT {
      | double Estado[5];
      | int Parametros[6];
      | unsigned int MedidasLaser[181];
      | } VIRTUAL_OUTPUT;
      mask DialogControl DescTextVar Prompt: This block is associated with the VREP model 'vrepModel_PioneerP3DX Hokuyo V2.2.ttm' available from the '\SAS TOOLS\Library V-REP Models & ScenesV1.0.0' folder. More Information on Help button.
      mask DialogControl Control2 Prompt: For multiple devices, set diferent number for each one.
      mask Display:
      | image('pioneer3dx_virtual.png')
      | color('red')
      | port_label('input',1,'actions')
      | port_label('input',2,'posture')
      | port_label('input',3,'parameters')
      | port_label('input',4,'parametersSend')
      | port_label('input',5,'postureSend')
      | color('white')
      | port_label('output',1,'state')
      | port_label('output',2,'parameters')
      | port_label('output',3,'laser')
  V-REP Pioneer STATIC : Subsystem  mask{id=6}
      mask prompts: id "Id Memoria Compartida"
      mask Display:
      | image('pioneer.jpg')
      | color('green')
      | port_label('input',  1, 'Posicion')
      | port_label('input',  2, 'Orientacion')
      | color ('blue')
      | port_label('output', 1, 'Posicion')
      | port_label('output', 2, 'Orientacion')
  V-REP static Quadricopter : Subsystem  mask{Id=1}
      mask prompts: Id "Device Number"
      mask Display:
      | image('vrepQuadricopter.png')
      | color('red');
      | port_label('input', 1, 'Position')
      | port_label('input', 2, 'Orientation')
      | color('blue');
      | port_label('output', 1, 'Position')
      | port_label('output', 2, 'Orientation')
      | port_label('output', 3, 'Linear Velocity')
      | port_label('output', 4, 'Angular Velocity')
  V-rep Open/Play/Stop : S-Function  FunctionName=vrepCommand  Parameters=scene  SFunctionDeploymentMode=off  EnableBusSupport=off  mask{scene='ejemplo.ttt'}
      mask prompts: scene "V-rep Scene Name"
      mask Display: image('vrepProEdu.png')
  V-rep Open/Play/Stop Synchronous MODE : S-Function  FunctionName=vrepSynchronous  Parameters=scene  SFunctionDeploymentMode=off  EnableBusSupport=off  mask{scene='ejemplo.ttt'}
      mask prompts: scene "V-rep Scene Name"
      mask Display: image('handshake.png')
  Visualizador : S-Function  FunctionName=visualizador  Parameters=1  SFunctionDeploymentMode=off  EnableBusSupport=off
      mask Display:
      | image('display_real.png')
      | color('red');
      | port_label('input', 1, 'Tiempo Muestreo')
      | port_label('input', 2, 'Tamaño')
      | port_label('input', 3, 'Imagen')
  WebCam : S-Function  FunctionName=webcam  Parameters=1  SFunctionDeploymentMode=off  EnableBusSupport=off
      mask Display:
      | image('webcam.png')
      | color('red');
      | port_label('output',1,'Imagen')
      | port_label('output',2,'Tamaño')
  plantilla IApp : Subsystem  mask{sys='IServer', shmControl='Server_ctr', shmInput='Server_In', shmOutput='Server_Out', shmInputVect=[68], shmOutputVect=[112], shmInputType=['C'], shmOutputType=['C'], period=100}
      mask prompts: sys "Program"; shmControl "Control Name"; shmInput "Input Name"; shmOutput "OutputName"; shmInputVect "Input Vector"; shmOutputVect "Output Vector"; shmInputType "Input Type"; shmOutputType "Output Type"; period "Period [us]"
      mask Display:
      | image('app.png')
      | color('blue');
      | port_label('input', 1, 'App Control')
      | port_label('input', 2, 'prams[char*]')
      | port_label('input', 3, 'Data In')
      | color('red');
      | port_label('output', 1, 'App ctr State')
      | port_label('output', 2, 'Error')
      | port_label('output', 3, 'Data Out')
  system : Subsystem  mask{nombre='memoriaEjemplo', dispositivo=10, vecE=[3 2 1], vecS=[4 5 6 156], tipoE=['f' 'I' 'c'], tipoS=['C' 'i' 'd' 'd'], ts=-1}
      mask prompts: nombre "Share Memory base name"; dispositivo "Device Number"; vecE "Sizes input vector"; vecS "Sizes output vector"; tipoE "Data Types Inputs"; tipoS "Data Types Outputs"; ts "Sample Time"
      mask Display: image('shmem.jpg')
  system clock UTC : S-Function  FunctionName=sysTime  SFunctionDeploymentMode=off  EnableBusSupport=off
      mask Display:
      | image('clock.jpg')
      | color('red');
      | port_label('output', 1, 'Year')
      | port_label('output', 2, 'Month')
      | port_label('output', 3, 'Week Day')
      | port_label('output', 4, 'Month Day')
      | port_label('output', 5, 'Hour')
      | port_label('output', 6, 'Minute')
      | port_label('output', 7, 'Second')
      | port_label('output', 8, 'Milliseconds')
